simulink model "large_model"  (Simulink 8.0) (continued)
== system large_model/Integrated_GPM2_4/GPM/Self_checking_pair_SSC2_pitch3 ==
  In1 : Inport
  In2 : Inport  Port=2
  In3 : Inport  Port=3
  In4 : Inport  Port=4
  In5 : Inport  Port=5
  In6 : Inport  Port=6
  In7 : Inport  Port=7
  In8 : Inport  Port=8
  Out : Outport
  wires:
  In1/1 -> Out/1

== system large_model/Integrated_GPM2_4/GPM/Self_checking_pair_SSC2_pitch4 ==
  In1 : Inport
  In2 : Inport  Port=2
  In3 : Inport  Port=3
  In4 : Inport  Port=4
  In5 : Inport  Port=5
  In6 : Inport  Port=6
  In7 : Inport  Port=7
  In8 : Inport  Port=8
  Out : Outport
  wires:
  In1/1 -> Out/1

== system large_model/Integrated_GPM2_4/GPM/Self_checking_pair_SSC2_roll1 ==
  In1 : Inport
  In2 : Inport  Port=2
  In3 : Inport  Port=3
  In4 : Inport  Port=4
  In5 : Inport  Port=5
  In6 : Inport  Port=6
  In7 : Inport  Port=7
  In8 : Inport  Port=8
  Out : Outport
  wires:
  In1/1 -> Out/1

== system large_model/Integrated_GPM2_4/GPM/Self_checking_pair_SSC2_roll2 ==
  In1 : Inport
  In2 : Inport  Port=2
  In3 : Inport  Port=3
  In4 : Inport  Port=4
  In5 : Inport  Port=5
  In6 : Inport  Port=6
  In7 : Inport  Port=7
  In8 : Inport  Port=8
  Out : Outport
  wires:
  In1/1 -> Out/1

== system large_model/Integrated_GPM2_4/GPM/Self_checking_pair_SSC2_roll3 ==
  In1 : Inport
  In2 : Inport  Port=2
  In3 : Inport  Port=3
  In4 : Inport  Port=4
  In5 : Inport  Port=5
  In6 : Inport  Port=6
  In7 : Inport  Port=7
  In8 : Inport  Port=8
  Out : Outport
  wires:
  In1/1 -> Out/1

== system large_model/Integrated_GPM2_4/GPM/Self_checking_pair_SSC2_roll4 ==
  In1 : Inport
  In2 : Inport  Port=2
  In3 : Inport  Port=3
  In4 : Inport  Port=4
  In5 : Inport  Port=5
  In6 : Inport  Port=6
  In7 : Inport  Port=7
  In8 : Inport  Port=8
  Out : Outport
  wires:
  In1/1 -> Out/1

== system large_model/Integrated_GPM2_4/GPM/Voter_ADS ==
  ADS1A : Inport
  ADS1B : Inport  Port=2
  ADS2A : Inport  Port=3
  ADS2B : Inport  Port=4
  ADS3A : Inport  Port=5
  ADS3B : Inport  Port=6
  ADS4A : Inport  Port=7
  ADS4B : Inport  Port=8
  Voter : Subsystem
  ADS : Outport
  wires:
  ADS4B/1 -> Voter/8(ADS4B_dat)
  ADS4A/1 -> Voter/7(ADS4A_dat)
  ADS3B/1 -> Voter/6(ADS3B_dat)
  ADS3A/1 -> Voter/5(ADS3A_dat)
  ADS2B/1 -> Voter/4(ADS2B_dat)
  ADS2A/1 -> Voter/3(ADS2A_dat)
  ADS1B/1 -> Voter/2(ADS1B_dat)
  Voter/1(ads_dat) -> ADS/1
  ADS1A/1 -> Voter/1(ADS1A_dat)
  [loss_2_4_flagged_2_unflagged] -> Voter/23(loss_2_4_flagged_2_unflagged)
  Voter/16(loss_2_4_flagged_2_unflagged_out) -> [loss_2_4_flagged_2_unflagged]
  [loss_3_4_unflagged] -> Voter/22(loss_3_4_unflagged)
  Voter/15(loss_3_4_unflagged_out) -> [loss_3_4_unflagged]
  [loss_2_4_flagged] -> Voter/21(loss_2_4_flagged)
  Voter/14(loss_2_4_flagged_out) -> [loss_2_4_flagged]
  [any_unflagged] -> Voter/20(any_unflagged)
  Voter/13(any_unflagged_out) -> [any_unflagged]
  [loss_ADS4_unflagged] -> Voter/19(loss_ADS4_unflagged)
  [loss_ADS3_unflagged] -> Voter/18(loss_ADS3_unflagged)
  Voter/12(loss_ADS4_unflagged_out) -> [loss_ADS4_unflagged]
  Voter/11(loss_ADS3_unflagged_out) -> [loss_ADS3_unflagged]
  [loss_ADS2_unflagged] -> Voter/17(loss_ADS2_unflagged)
  Voter/10(loss_ADS2_unflagged_out) -> [loss_ADS2_unflagged]
  [loss_ADS1_unflagged] -> Voter/16(loss_ADS1_unflagged)
  Voter/9(loss_ADS1_unflagged_out) -> [loss_ADS1_unflagged]
  [loss_ADS4_flagged] -> Voter/15(loss_ADS4_flagged)
  Voter/8(loss_ADS4_flagged_out) -> [loss_ADS4_flagged]
  [loss_ADS3_flagged] -> Voter/14(loss_ADS3_flagged)
  Voter/7(loss_ADS3_flagged_out) -> [loss_ADS3_flagged]
  [loss_ADS2_flagged] -> Voter/13(loss_ADS2_flagged)
  Voter/6(loss_ADS2_flagged_out) -> [loss_ADS2_flagged]
  [loss_ADS1_flagged] -> Voter/12(loss_ADS1_flagged)
  Voter/5(loss_ADS1_flagged_out) -> [loss_ADS1_flagged]
  [loss_2_4_flagged_1_unflagged] -> Voter/11(loss_2_4_flagged_1_unflagged)
  Voter/4(loss_2_4_flagged_1_unflagged_out) -> [loss_2_4_flagged_1_unflagged]
  [loss_2_4_unflagged] -> Voter/10(loss_2_4_unflagged)
  Voter/3(loss_2_4_unflagged_out) -> [loss_2_4_unflagged]
  Voter/2(loss_3_4_flagged_out) -> [loss_3_4_flagged]
  [loss_3_4_flagged] -> Voter/9(loss_3_4_flagged)

== system large_model/GPM_IO_1/Integrated_GPM0/End_System/ES Hardware ==
  In1 : Inport
  In2 : Inport  Port=2
  PWR : Inport  Port=3
  Out1 : Outport
  Out2 : Outport  Port=2
  wires:
  In2/1 -> Out2/1
  In1/1 -> Out1/1

== system large_model/GPM_IO_1/Integrated_GPM0/GPM/GPM Hardware ==
  In : Inport
  PWR_In : Inport  Port=2
  Out : Outport
  wires:
  In/1 -> Out/1

== system large_model/GPM_IO_1/Integrated_GPM0/GPM/PWR ==
  In : Inport
  Out : Outport
  wires:
  In/1 -> Out/1

== system large_model/GPM_IO_2/Integrated_GPM0/End_System/ES Hardware ==
  In1 : Inport
  In2 : Inport  Port=2
  PWR : Inport  Port=3
  Out1 : Outport
  Out2 : Outport  Port=2
  wires:
  In2/1 -> Out2/1
  In1/1 -> Out1/1

== system large_model/GPM_IO_2/Integrated_GPM0/GPM/GPM Hardware ==
  In : Inport
  PWR_In : Inport  Port=2
  Out : Outport
  wires:
  In/1 -> Out/1

== system large_model/GPM_IO_2/Integrated_GPM0/GPM/PWR ==
  In : Inport
  Out : Outport
  wires:
  In/1 -> Out/1

== system large_model/GPM_IO_3/Integrated_GPM0/End_System/ES Hardware ==
  In1 : Inport
  In2 : Inport  Port=2
  PWR : Inport  Port=3
  Out1 : Outport
  Out2 : Outport  Port=2
  wires:
  In2/1 -> Out2/1
  In1/1 -> Out1/1

== system large_model/GPM_IO_3/Integrated_GPM0/GPM/GPM Hardware ==
  In : Inport
  PWR_In : Inport  Port=2
  Out : Outport
  wires:
  In/1 -> Out/1

== system large_model/GPM_IO_3/Integrated_GPM0/GPM/PWR ==
  In : Inport
  Out : Outport
  wires:
  In/1 -> Out/1

== system large_model/GPM_IO_4/Integrated_GPM0/End_System/ES Hardware ==
  In1 : Inport
  In2 : Inport  Port=2
  PWR : Inport  Port=3
  Out1 : Outport
  Out2 : Outport  Port=2
  wires:
  In2/1 -> Out2/1
  In1/1 -> Out1/1

== system large_model/GPM_IO_4/Integrated_GPM0/GPM/GPM Hardware ==
  In : Inport
  PWR_In : Inport  Port=2
  Out : Outport
  wires:
  In/1 -> Out/1

== system large_model/GPM_IO_4/Integrated_GPM0/GPM/PWR ==
  In : Inport
  Out : Outport
  wires:
  In/1 -> Out/1

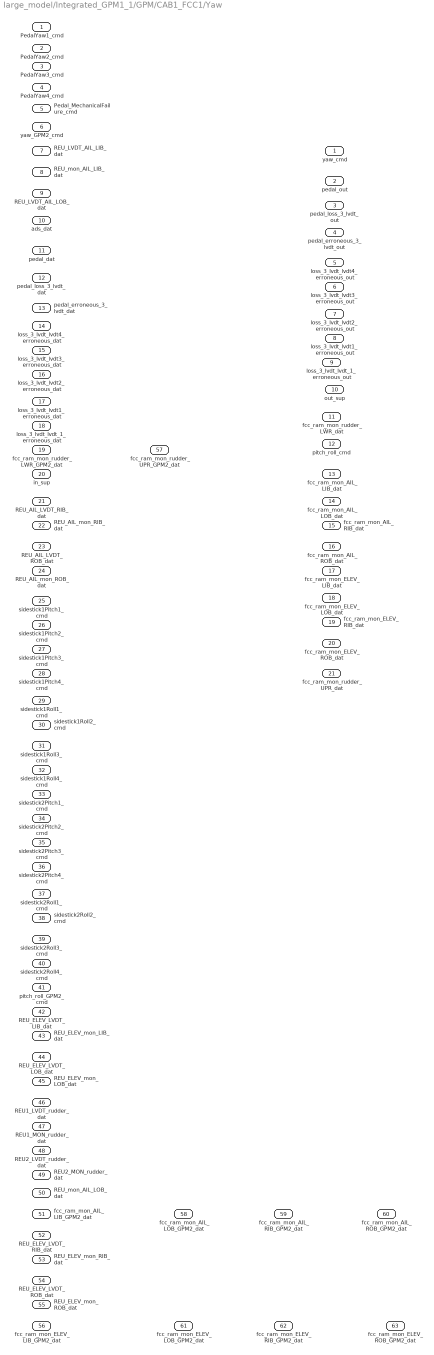
== system large_model/Integrated_GPM1_1/GPM/CAB1_FCC1/Yaw ==
  PedalYaw1_cmd : Inport
  PedalYaw2_cmd : Inport  Port=2
  PedalYaw3_cmd : Inport  Port=3
  PedalYaw4_cmd : Inport  Port=4
  Pedal_MechanicalFailure_cmd : Inport  Port=5
  yaw_GPM2_cmd : Inport  Port=6
  REU_LVDT_AIL_LIB_dat : Inport  Port=7
  REU_mon_AIL_LIB_dat : Inport  Port=8
  REU_LVDT_AIL_LOB_dat : Inport  Port=9
  ads_dat : Inport  Port=10
  pedal_dat : Inport  Port=11
  pedal_loss_3_lvdt_dat : Inport  Port=12
  pedal_erroneous_3_lvdt_dat : Inport  Port=13
  loss_3_lvdt_lvdt4_erroneous_dat : Inport  Port=14
  loss_3_lvdt_lvdt3_erroneous_dat : Inport  Port=15
  loss_3_lvdt_lvdt2_erroneous_dat : Inport  Port=16
  loss_3_lvdt_lvdt1_erroneous_dat : Inport  Port=17
  loss_3_lvdt_lvdt_1_erroneous_dat : Inport  Port=18
  fcc_ram_mon_rudder_LWR_GPM2_dat : Inport  Port=19
  in_sup : Inport  Port=20
  REU_AIL_LVDT_RIB_dat : Inport  Port=21
  REU_AIL_mon_RIB_dat : Inport  Port=22
  REU_AIL_LVDT_ROB_dat : Inport  Port=23
  REU_AIL_mon_ROB_dat : Inport  Port=24
  sidestick1Pitch1_cmd : Inport  Port=25
  sidestick1Pitch2_cmd : Inport  Port=26
  sidestick1Pitch3_cmd : Inport  Port=27
  sidestick1Pitch4_cmd : Inport  Port=28
  sidestick1Roll1_cmd : Inport  Port=29
  sidestick1Roll2_cmd : Inport  Port=30
  sidestick1Roll3_cmd : Inport  Port=31
  sidestick1Roll4_cmd : Inport  Port=32
  sidestick2Pitch1_cmd : Inport  Port=33
  sidestick2Pitch2_cmd : Inport  Port=34
  sidestick2Pitch3_cmd : Inport  Port=35
  sidestick2Pitch4_cmd : Inport  Port=36
  sidestick2Roll1_cmd : Inport  Port=37
  sidestick2Roll2_cmd : Inport  Port=38
  sidestick2Roll3_cmd : Inport  Port=39
  sidestick2Roll4_cmd : Inport  Port=40
  pitch_roll_GPM2_cmd : Inport  Port=41
  REU_ELEV_LVDT_LIB_dat : Inport  Port=42
  REU_ELEV_mon_LIB_dat : Inport  Port=43
  REU_ELEV_LVDT_LOB_dat : Inport  Port=44
  REU_ELEV_mon_LOB_dat : Inport  Port=45
  REU1_LVDT_rudder_dat : Inport  Port=46
  REU1_MON_rudder_dat : Inport  Port=47
  REU2_LVDT_rudder_dat : Inport  Port=48
  REU2_MON_rudder_dat : Inport  Port=49
  REU_mon_AIL_LOB_dat : Inport  Port=50
  fcc_ram_mon_AIL_LIB_GPM2_dat : Inport  Port=51
  REU_ELEV_LVDT_RIB_dat : Inport  Port=52
  REU_ELEV_mon_RIB_dat : Inport  Port=53
  REU_ELEV_LVDT_ROB_dat : Inport  Port=54
  REU_ELEV_mon_ROB_dat : Inport  Port=55
  fcc_ram_mon_ELEV_LIB_GPM2_dat : Inport  Port=56
  fcc_ram_mon_rudder_UPR_GPM2_dat : Inport  Port=57
  fcc_ram_mon_AIL_LOB_GPM2_dat : Inport  Port=58
  fcc_ram_mon_AIL_RIB_GPM2_dat : Inport  Port=59
  fcc_ram_mon_AIL_ROB_GPM2_dat : Inport  Port=60
  fcc_ram_mon_ELEV_LOB_GPM2_dat : Inport  Port=61
  fcc_ram_mon_ELEV_RIB_GPM2_dat : Inport  Port=62
  fcc_ram_mon_ELEV_ROB_GPM2_dat : Inport  Port=63
  yaw_cmd : Outport
  pedal_out : Outport  Port=2
  pedal_loss_3_lvdt_out : Outport  Port=3
  pedal_erroneous_3_lvdt_out : Outport  Port=4
  loss_3_lvdt_lvdt4_erroneous_out : Outport  Port=5
  loss_3_lvdt_lvdt3_erroneous_out : Outport  Port=6
  loss_3_lvdt_lvdt2_erroneous_out : Outport  Port=7
  loss_3_lvdt_lvdt1_erroneous_out : Outport  Port=8
  loss_3_lvdt_lvdt_1_erroneous_out : Outport  Port=9
  out_sup : Outport  Port=10
  fcc_ram_mon_rudder_LWR_dat : Outport  Port=11
  pitch_roll_cmd : Outport  Port=12
  fcc_ram_mon_AIL_LIB_dat : Outport  Port=13
  fcc_ram_mon_AIL_LOB_dat : Outport  Port=14
  fcc_ram_mon_AIL_RIB_dat : Outport  Port=15
  fcc_ram_mon_AIL_ROB_dat : Outport  Port=16
  fcc_ram_mon_ELEV_LIB_dat : Outport  Port=17
  fcc_ram_mon_ELEV_LOB_dat : Outport  Port=18
  fcc_ram_mon_ELEV_RIB_dat : Outport  Port=19
  fcc_ram_mon_ELEV_ROB_dat : Outport  Port=20
  fcc_ram_mon_rudder_UPR_dat : Outport  Port=21

== system large_model/Integrated_GPM1_1/GPM/Voter_ADS/Voter ==
  ADS1A_dat : Inport
  ADS1B_dat : Inport  Port=2
  ADS2A_dat : Inport  Port=3
  ADS2B_dat : Inport  Port=4
  ADS3A_dat : Inport  Port=5
  ADS3B_dat : Inport  Port=6
  ADS4A_dat : Inport  Port=7
  ADS4B_dat : Inport  Port=8
  loss_3_4_flagged : Inport  Port=9
  loss_2_4_unflagged : Inport  Port=10
  loss_2_4_flagged_1_unflagged : Inport  Port=11
  loss_ADS1_flagged : Inport  Port=12
  loss_ADS2_flagged : Inport  Port=13
  loss_ADS3_flagged : Inport  Port=14
  loss_ADS4_flagged : Inport  Port=15
  loss_ADS1_unflagged : Inport  Port=16
  loss_ADS2_unflagged : Inport  Port=17
  loss_ADS3_unflagged : Inport  Port=18
  loss_ADS4_unflagged : Inport  Port=19
  any_unflagged : Inport  Port=20
  loss_2_4_flagged : Inport  Port=21
  loss_3_4_unflagged : Inport  Port=22
  loss_2_4_flagged_2_unflagged : Inport  Port=23
  ads_dat : Outport
  loss_3_4_flagged_out : Outport  Port=2
  loss_2_4_unflagged_out : Outport  Port=3
  loss_2_4_flagged_1_unflagged_out : Outport  Port=4
  loss_ADS1_flagged_out : Outport  Port=5
  loss_ADS2_flagged_out : Outport  Port=6
  loss_ADS3_flagged_out : Outport  Port=7
  loss_ADS4_flagged_out : Outport  Port=8
  loss_ADS1_unflagged_out : Outport  Port=9
  loss_ADS2_unflagged_out : Outport  Port=10
  loss_ADS3_unflagged_out : Outport  Port=11
  loss_ADS4_unflagged_out : Outport  Port=12
  any_unflagged_out : Outport  Port=13
  loss_2_4_flagged_out : Outport  Port=14
  loss_3_4_unflagged_out : Outport  Port=15
  loss_2_4_flagged_2_unflagged_out : Outport  Port=16

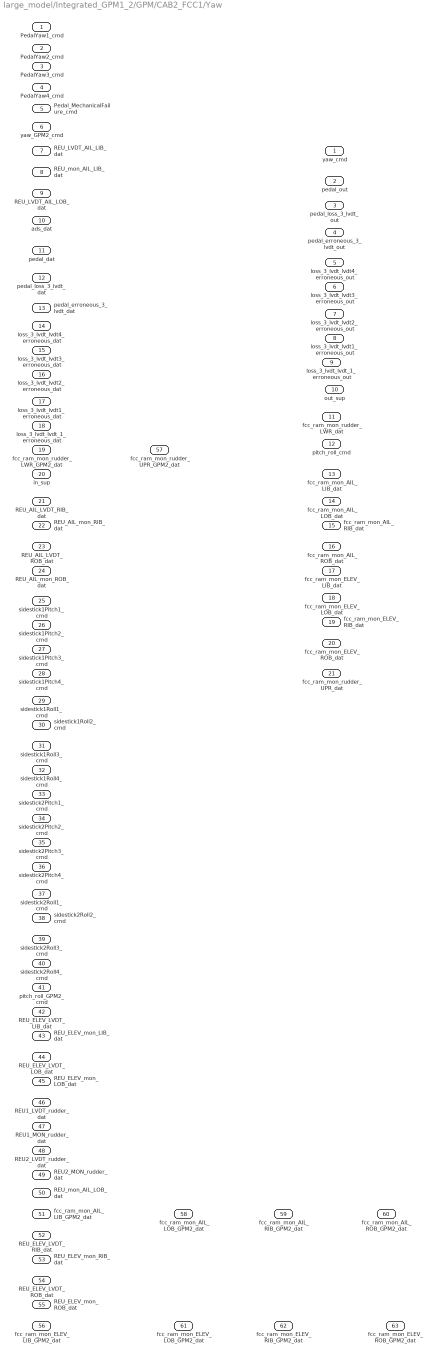
== system large_model/Integrated_GPM1_2/GPM/CAB2_FCC1/Yaw ==
  PedalYaw1_cmd : Inport
  PedalYaw2_cmd : Inport  Port=2
  PedalYaw3_cmd : Inport  Port=3
  PedalYaw4_cmd : Inport  Port=4
  Pedal_MechanicalFailure_cmd : Inport  Port=5
  yaw_GPM2_cmd : Inport  Port=6
  REU_LVDT_AIL_LIB_dat : Inport  Port=7
  REU_mon_AIL_LIB_dat : Inport  Port=8
  REU_LVDT_AIL_LOB_dat : Inport  Port=9
  ads_dat : Inport  Port=10
  pedal_dat : Inport  Port=11
  pedal_loss_3_lvdt_dat : Inport  Port=12
  pedal_erroneous_3_lvdt_dat : Inport  Port=13
  loss_3_lvdt_lvdt4_erroneous_dat : Inport  Port=14
  loss_3_lvdt_lvdt3_erroneous_dat : Inport  Port=15
  loss_3_lvdt_lvdt2_erroneous_dat : Inport  Port=16
  loss_3_lvdt_lvdt1_erroneous_dat : Inport  Port=17
  loss_3_lvdt_lvdt_1_erroneous_dat : Inport  Port=18
  fcc_ram_mon_rudder_LWR_GPM2_dat : Inport  Port=19
  in_sup : Inport  Port=20
  REU_AIL_LVDT_RIB_dat : Inport  Port=21
  REU_AIL_mon_RIB_dat : Inport  Port=22
  REU_AIL_LVDT_ROB_dat : Inport  Port=23
  REU_AIL_mon_ROB_dat : Inport  Port=24
  sidestick1Pitch1_cmd : Inport  Port=25
  sidestick1Pitch2_cmd : Inport  Port=26
  sidestick1Pitch3_cmd : Inport  Port=27
  sidestick1Pitch4_cmd : Inport  Port=28
  sidestick1Roll1_cmd : Inport  Port=29
  sidestick1Roll2_cmd : Inport  Port=30
  sidestick1Roll3_cmd : Inport  Port=31
  sidestick1Roll4_cmd : Inport  Port=32
  sidestick2Pitch1_cmd : Inport  Port=33
  sidestick2Pitch2_cmd : Inport  Port=34
  sidestick2Pitch3_cmd : Inport  Port=35
  sidestick2Pitch4_cmd : Inport  Port=36
  sidestick2Roll1_cmd : Inport  Port=37
  sidestick2Roll2_cmd : Inport  Port=38
  sidestick2Roll3_cmd : Inport  Port=39
  sidestick2Roll4_cmd : Inport  Port=40
  pitch_roll_GPM2_cmd : Inport  Port=41
  REU_ELEV_LVDT_LIB_dat : Inport  Port=42
  REU_ELEV_mon_LIB_dat : Inport  Port=43
  REU_ELEV_LVDT_LOB_dat : Inport  Port=44
  REU_ELEV_mon_LOB_dat : Inport  Port=45
  REU1_LVDT_rudder_dat : Inport  Port=46
  REU1_MON_rudder_dat : Inport  Port=47
  REU2_LVDT_rudder_dat : Inport  Port=48
  REU2_MON_rudder_dat : Inport  Port=49
  REU_mon_AIL_LOB_dat : Inport  Port=50
  fcc_ram_mon_AIL_LIB_GPM2_dat : Inport  Port=51
  REU_ELEV_LVDT_RIB_dat : Inport  Port=52
  REU_ELEV_mon_RIB_dat : Inport  Port=53
  REU_ELEV_LVDT_ROB_dat : Inport  Port=54
  REU_ELEV_mon_ROB_dat : Inport  Port=55
  fcc_ram_mon_ELEV_LIB_GPM2_dat : Inport  Port=56
  fcc_ram_mon_rudder_UPR_GPM2_dat : Inport  Port=57
  fcc_ram_mon_AIL_LOB_GPM2_dat : Inport  Port=58
  fcc_ram_mon_AIL_RIB_GPM2_dat : Inport  Port=59
  fcc_ram_mon_AIL_ROB_GPM2_dat : Inport  Port=60
  fcc_ram_mon_ELEV_LOB_GPM2_dat : Inport  Port=61
  fcc_ram_mon_ELEV_RIB_GPM2_dat : Inport  Port=62
  fcc_ram_mon_ELEV_ROB_GPM2_dat : Inport  Port=63
  yaw_cmd : Outport
  pedal_out : Outport  Port=2
  pedal_loss_3_lvdt_out : Outport  Port=3
  pedal_erroneous_3_lvdt_out : Outport  Port=4
  loss_3_lvdt_lvdt4_erroneous_out : Outport  Port=5
  loss_3_lvdt_lvdt3_erroneous_out : Outport  Port=6
  loss_3_lvdt_lvdt2_erroneous_out : Outport  Port=7
  loss_3_lvdt_lvdt1_erroneous_out : Outport  Port=8
  loss_3_lvdt_lvdt_1_erroneous_out : Outport  Port=9
  out_sup : Outport  Port=10
  fcc_ram_mon_rudder_LWR_dat : Outport  Port=11
  pitch_roll_cmd : Outport  Port=12
  fcc_ram_mon_AIL_LIB_dat : Outport  Port=13
  fcc_ram_mon_AIL_LOB_dat : Outport  Port=14
  fcc_ram_mon_AIL_RIB_dat : Outport  Port=15
  fcc_ram_mon_AIL_ROB_dat : Outport  Port=16
  fcc_ram_mon_ELEV_LIB_dat : Outport  Port=17
  fcc_ram_mon_ELEV_LOB_dat : Outport  Port=18
  fcc_ram_mon_ELEV_RIB_dat : Outport  Port=19
  fcc_ram_mon_ELEV_ROB_dat : Outport  Port=20
  fcc_ram_mon_rudder_UPR_dat : Outport  Port=21

== system large_model/Integrated_GPM1_2/GPM/Voter_ADS/Voter ==
  ADS1A_dat : Inport
  ADS1B_dat : Inport  Port=2
  ADS2A_dat : Inport  Port=3
  ADS2B_dat : Inport  Port=4
  ADS3A_dat : Inport  Port=5
  ADS3B_dat : Inport  Port=6
  ADS4A_dat : Inport  Port=7
  ADS4B_dat : Inport  Port=8
  loss_3_4_flagged : Inport  Port=9
  loss_2_4_unflagged : Inport  Port=10
  loss_2_4_flagged_1_unflagged : Inport  Port=11
  loss_ADS1_flagged : Inport  Port=12
  loss_ADS2_flagged : Inport  Port=13
  loss_ADS3_flagged : Inport  Port=14
  loss_ADS4_flagged : Inport  Port=15
  loss_ADS1_unflagged : Inport  Port=16
  loss_ADS2_unflagged : Inport  Port=17
  loss_ADS3_unflagged : Inport  Port=18
  loss_ADS4_unflagged : Inport  Port=19
  any_unflagged : Inport  Port=20
  loss_2_4_flagged : Inport  Port=21
  loss_3_4_unflagged : Inport  Port=22
  loss_2_4_flagged_2_unflagged : Inport  Port=23
  ads_dat : Outport
  loss_3_4_flagged_out : Outport  Port=2
  loss_2_4_unflagged_out : Outport  Port=3
  loss_2_4_flagged_1_unflagged_out : Outport  Port=4
  loss_ADS1_flagged_out : Outport  Port=5
  loss_ADS2_flagged_out : Outport  Port=6
  loss_ADS3_flagged_out : Outport  Port=7
  loss_ADS4_flagged_out : Outport  Port=8
  loss_ADS1_unflagged_out : Outport  Port=9
  loss_ADS2_unflagged_out : Outport  Port=10
  loss_ADS3_unflagged_out : Outport  Port=11
  loss_ADS4_unflagged_out : Outport  Port=12
  any_unflagged_out : Outport  Port=13
  loss_2_4_flagged_out : Outport  Port=14
  loss_3_4_unflagged_out : Outport  Port=15
  loss_2_4_flagged_2_unflagged_out : Outport  Port=16

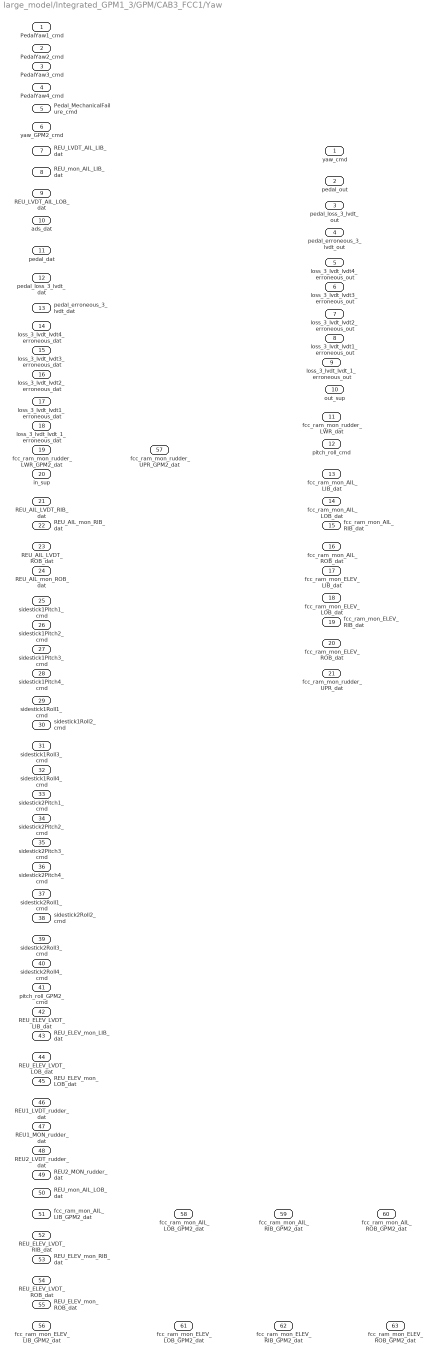
== system large_model/Integrated_GPM1_3/GPM/CAB3_FCC1/Yaw ==
  PedalYaw1_cmd : Inport
  PedalYaw2_cmd : Inport  Port=2
  PedalYaw3_cmd : Inport  Port=3
  PedalYaw4_cmd : Inport  Port=4
  Pedal_MechanicalFailure_cmd : Inport  Port=5
  yaw_GPM2_cmd : Inport  Port=6
  REU_LVDT_AIL_LIB_dat : Inport  Port=7
  REU_mon_AIL_LIB_dat : Inport  Port=8
  REU_LVDT_AIL_LOB_dat : Inport  Port=9
  ads_dat : Inport  Port=10
  pedal_dat : Inport  Port=11
  pedal_loss_3_lvdt_dat : Inport  Port=12
  pedal_erroneous_3_lvdt_dat : Inport  Port=13
  loss_3_lvdt_lvdt4_erroneous_dat : Inport  Port=14
  loss_3_lvdt_lvdt3_erroneous_dat : Inport  Port=15
  loss_3_lvdt_lvdt2_erroneous_dat : Inport  Port=16
  loss_3_lvdt_lvdt1_erroneous_dat : Inport  Port=17
  loss_3_lvdt_lvdt_1_erroneous_dat : Inport  Port=18
  fcc_ram_mon_rudder_LWR_GPM2_dat : Inport  Port=19
  in_sup : Inport  Port=20
  REU_AIL_LVDT_RIB_dat : Inport  Port=21
  REU_AIL_mon_RIB_dat : Inport  Port=22
  REU_AIL_LVDT_ROB_dat : Inport  Port=23
  REU_AIL_mon_ROB_dat : Inport  Port=24
  sidestick1Pitch1_cmd : Inport  Port=25
  sidestick1Pitch2_cmd : Inport  Port=26
  sidestick1Pitch3_cmd : Inport  Port=27
  sidestick1Pitch4_cmd : Inport  Port=28
  sidestick1Roll1_cmd : Inport  Port=29
  sidestick1Roll2_cmd : Inport  Port=30
  sidestick1Roll3_cmd : Inport  Port=31
  sidestick1Roll4_cmd : Inport  Port=32
  sidestick2Pitch1_cmd : Inport  Port=33
  sidestick2Pitch2_cmd : Inport  Port=34
  sidestick2Pitch3_cmd : Inport  Port=35
  sidestick2Pitch4_cmd : Inport  Port=36
  sidestick2Roll1_cmd : Inport  Port=37
  sidestick2Roll2_cmd : Inport  Port=38
  sidestick2Roll3_cmd : Inport  Port=39
  sidestick2Roll4_cmd : Inport  Port=40
  pitch_roll_GPM2_cmd : Inport  Port=41
  REU_ELEV_LVDT_LIB_dat : Inport  Port=42
  REU_ELEV_mon_LIB_dat : Inport  Port=43
  REU_ELEV_LVDT_LOB_dat : Inport  Port=44
  REU_ELEV_mon_LOB_dat : Inport  Port=45
  REU1_LVDT_rudder_dat : Inport  Port=46
  REU1_MON_rudder_dat : Inport  Port=47
  REU2_LVDT_rudder_dat : Inport  Port=48
  REU2_MON_rudder_dat : Inport  Port=49
  REU_mon_AIL_LOB_dat : Inport  Port=50
  fcc_ram_mon_AIL_LIB_GPM2_dat : Inport  Port=51
  REU_ELEV_LVDT_RIB_dat : Inport  Port=52
  REU_ELEV_mon_RIB_dat : Inport  Port=53
  REU_ELEV_LVDT_ROB_dat : Inport  Port=54
  REU_ELEV_mon_ROB_dat : Inport  Port=55
  fcc_ram_mon_ELEV_LIB_GPM2_dat : Inport  Port=56
  fcc_ram_mon_rudder_UPR_GPM2_dat : Inport  Port=57
  fcc_ram_mon_AIL_LOB_GPM2_dat : Inport  Port=58
  fcc_ram_mon_AIL_RIB_GPM2_dat : Inport  Port=59
  fcc_ram_mon_AIL_ROB_GPM2_dat : Inport  Port=60
  fcc_ram_mon_ELEV_LOB_GPM2_dat : Inport  Port=61
  fcc_ram_mon_ELEV_RIB_GPM2_dat : Inport  Port=62
  fcc_ram_mon_ELEV_ROB_GPM2_dat : Inport  Port=63
  yaw_cmd : Outport
  pedal_out : Outport  Port=2
  pedal_loss_3_lvdt_out : Outport  Port=3
  pedal_erroneous_3_lvdt_out : Outport  Port=4
  loss_3_lvdt_lvdt4_erroneous_out : Outport  Port=5
  loss_3_lvdt_lvdt3_erroneous_out : Outport  Port=6
  loss_3_lvdt_lvdt2_erroneous_out : Outport  Port=7
  loss_3_lvdt_lvdt1_erroneous_out : Outport  Port=8
  loss_3_lvdt_lvdt_1_erroneous_out : Outport  Port=9
  out_sup : Outport  Port=10
  fcc_ram_mon_rudder_LWR_dat : Outport  Port=11
  pitch_roll_cmd : Outport  Port=12
  fcc_ram_mon_AIL_LIB_dat : Outport  Port=13
  fcc_ram_mon_AIL_LOB_dat : Outport  Port=14
  fcc_ram_mon_AIL_RIB_dat : Outport  Port=15
  fcc_ram_mon_AIL_ROB_dat : Outport  Port=16
  fcc_ram_mon_ELEV_LIB_dat : Outport  Port=17
  fcc_ram_mon_ELEV_LOB_dat : Outport  Port=18
  fcc_ram_mon_ELEV_RIB_dat : Outport  Port=19
  fcc_ram_mon_ELEV_ROB_dat : Outport  Port=20
  fcc_ram_mon_rudder_UPR_dat : Outport  Port=21

== system large_model/Integrated_GPM1_3/GPM/Voter_ADS/Voter ==
  ADS1A_dat : Inport
  ADS1B_dat : Inport  Port=2
  ADS2A_dat : Inport  Port=3
  ADS2B_dat : Inport  Port=4
  ADS3A_dat : Inport  Port=5
  ADS3B_dat : Inport  Port=6
  ADS4A_dat : Inport  Port=7
  ADS4B_dat : Inport  Port=8
  loss_3_4_flagged : Inport  Port=9
  loss_2_4_unflagged : Inport  Port=10
  loss_2_4_flagged_1_unflagged : Inport  Port=11
  loss_ADS1_flagged : Inport  Port=12
  loss_ADS2_flagged : Inport  Port=13
  loss_ADS3_flagged : Inport  Port=14
  loss_ADS4_flagged : Inport  Port=15
  loss_ADS1_unflagged : Inport  Port=16
  loss_ADS2_unflagged : Inport  Port=17
  loss_ADS3_unflagged : Inport  Port=18
  loss_ADS4_unflagged : Inport  Port=19
  any_unflagged : Inport  Port=20
  loss_2_4_flagged : Inport  Port=21
  loss_3_4_unflagged : Inport  Port=22
  loss_2_4_flagged_2_unflagged : Inport  Port=23
  ads_dat : Outport
  loss_3_4_flagged_out : Outport  Port=2
  loss_2_4_unflagged_out : Outport  Port=3
  loss_2_4_flagged_1_unflagged_out : Outport  Port=4
  loss_ADS1_flagged_out : Outport  Port=5
  loss_ADS2_flagged_out : Outport  Port=6
  loss_ADS3_flagged_out : Outport  Port=7
  loss_ADS4_flagged_out : Outport  Port=8
  loss_ADS1_unflagged_out : Outport  Port=9
  loss_ADS2_unflagged_out : Outport  Port=10
  loss_ADS3_unflagged_out : Outport  Port=11
  loss_ADS4_unflagged_out : Outport  Port=12
  any_unflagged_out : Outport  Port=13
  loss_2_4_flagged_out : Outport  Port=14
  loss_3_4_unflagged_out : Outport  Port=15
  loss_2_4_flagged_2_unflagged_out : Outport  Port=16
